simulink library "lib_all"  (Simulink 7.6)
settings: Solver=ode45  SolverMode=SingleTasking  StartTime=0.0  StopTime=10.0  RelTol=1e-3
values from neighbouring files (symbols this model uses):
  map_lib_03 = [6.8679 7.0159 7.4571 8.1849 9.1892 10.456 11.97 13.71 15.655 17.781 20.063 22.474 ... (803 elements, 73x11)]    [scr_simdata.mat]    (also in scr_simdata_all.mat)
  map_lib_06 = [2.5784 2.6727 2.9588 3.4453 4.1444 5.068 6.226 7.6242 9.2634 11.139 13.24 15.552 ... (803 elements, 73x11)]    [scr_simdata.mat]    (also in scr_simdata_all.mat)
  map_lib_09 = [0.0025784 0.0026727 0.0029588 0.0034453 0.0041444 0.005068 0.006226 0.0076242 0.0092634 0.011139 0.01324 0.015552 ... (803 elements, 73x11)]    [scr_simdata.mat]    (also in scr_simdata_all.mat)
  map_lib_10 = [2.74 2.75 2.76 2.77 2.78 2.79 2.8 2.81 2.82 2.83 2.84 2.85 ... (81 elements, 1x81)]    [scr_simdata.mat]    (also in scr_simdata_all.mat)
  map_lib_11 = [1.1983 1.2027 1.207 1.2113 1.2156 1.2198 1.2241 1.2283 1.2326 1.2368 1.241 1.2451 ... (81 elements, 1x81)]    [scr_simdata.mat]    (also in scr_simdata_all.mat)
  map_lib_12 = [2.74 2.75 2.76 2.77 2.78 2.79 2.8 2.81 2.82 2.83 2.84 2.85 ... (81 elements, 1x81)]    [scr_simdata.mat]    (also in scr_simdata_all.mat)
  map_lib_13 = [1.74 1.75 1.76 1.77 1.78 1.79 1.8 1.81 1.82 1.83 1.84 1.85 ... (81 elements, 1x81)]    [scr_simdata.mat]    (also in scr_simdata_all.mat)
  map_lib_16 = [0.00048828125 1.6159 1.8962 2.0821 2.2251 2.3426 2.4433 2.5318 2.611 2.683 2.749 2.8101 ... (1419 elements, 129x11)]    [scr_simdata.mat]    (also in scr_simdata_all.mat)
  map_mr2_01 = [0 800 1600 2400 3200 4000]    [scr_simdata.mat]    (also in scr_simdata_all.mat)
  map_mr2_02 = [1.32 1.32 1.33 1.34 1.36 1.38]    [scr_simdata.mat]    (also in scr_simdata_all.mat)
  map_mr2_03 = [0 800 1600 2400 3200 4000]    [scr_simdata.mat]    (also in scr_simdata_all.mat)
  map_mr2_04 = [1.32 1.32 1.33 1.34 1.36 1.38]    [scr_simdata.mat]    (also in scr_simdata_all.mat)
  map_mr2_05 = [0 800 1600 2400 3200 4000]    [scr_simdata.mat]    (also in scr_simdata_all.mat)
  map_mr2_06 = [1.32 1.32 1.33 1.34 1.36 1.38]    [scr_simdata.mat]    (also in scr_simdata_all.mat)
  Parameter1 = 6    [scr_simdata.mat]    (also in scr_simdata_all.mat)
  Parameter101 = 30    [scr_simdata.mat]    (also in scr_simdata_all.mat)
  Parameter102 = 12    [scr_simdata.mat]    (also in scr_simdata_all.mat)
  Parameter103 = -240    [scr_simdata.mat]    (also in scr_simdata_all.mat)
  Parameter108 = 48    [scr_simdata.mat]    (also in scr_simdata_all.mat)
  Parameter120 = 16    [scr_simdata.mat]    (also in scr_simdata_all.mat)
  Parameter121 = 87    [scr_simdata.mat]    (also in scr_simdata_all.mat)
  Parameter123 = 78    [scr_simdata.mat]    (also in scr_simdata_all.mat)
  Parameter124 = 60    [scr_simdata.mat]    (also in scr_simdata_all.mat)
  Parameter125 = -480    [scr_simdata.mat]    (also in scr_simdata_all.mat)
  Parameter130 = 96    [scr_simdata.mat]    (also in scr_simdata_all.mat)
  Parameter131 = 3    [scr_simdata.mat]    (also in scr_simdata_all.mat)
  Parameter144 = 3    [scr_simdata.mat]    (also in scr_simdata_all.mat)
  Parameter18 = 128    [scr_simdata.mat]    (also in scr_simdata_all.mat)
  Parameter19 = 16    [scr_simdata.mat]    (also in scr_simdata_all.mat)
  Parameter2 = 12    [scr_simdata.mat]    (also in scr_simdata_all.mat)
  Parameter20 = 7    [scr_simdata.mat]    (also in scr_simdata_all.mat)
  Parameter21 = 4    [scr_simdata.mat]    (also in scr_simdata_all.mat)
  Parameter3 = 18    [scr_simdata.mat]    (also in scr_simdata_all.mat)
  Parameter32 = 1    [scr_simdata.mat]    (also in scr_simdata_all.mat)
  Parameter34 = 24    [scr_simdata.mat]    (also in scr_simdata_all.mat)
  Parameter4 = 138    [scr_simdata.mat]    (also in scr_simdata_all.mat)
  Parameter42 = 48    [scr_simdata.mat]    (also in scr_simdata_all.mat)
  Parameter5 = 135    [scr_simdata.mat]    (also in scr_simdata_all.mat)
  Parameter55 = 0    [scr_simdata.mat]    (also in scr_simdata_all.mat)
  Parameter56 = 0    [scr_simdata.mat]    (also in scr_simdata_all.mat)
  Parameter57 = 1    [scr_simdata.mat]    (also in scr_simdata_all.mat)
  Parameter58 = 1    [scr_simdata.mat]    (also in scr_simdata_all.mat)
  Parameter59 = 2    [scr_simdata.mat]    (also in scr_simdata_all.mat)
  Parameter6 = 132    [scr_simdata.mat]    (also in scr_simdata_all.mat)
  Parameter60 = 2    [scr_simdata.mat]    (also in scr_simdata_all.mat)
  Parameter63 = 144    [scr_simdata.mat]    (also in scr_simdata_all.mat)
  Parameter7 = 126    [scr_simdata.mat]    (also in scr_simdata_all.mat)
  Parameter76 = 64    [scr_simdata.mat]    (also in scr_simdata_all.mat)
  Parameter77 = 135    [scr_simdata.mat]    (also in scr_simdata_all.mat)
  Parameter79 = 126    [scr_simdata.mat]    (also in scr_simdata_all.mat)
  Parameter80 = 108    [scr_simdata.mat]    (also in scr_simdata_all.mat)
  Parameter81 = 0    [scr_simdata.mat]    (also in scr_simdata_all.mat)
  Parameter86 = 0    [scr_simdata.mat]    (also in scr_simdata_all.mat)
  Parameter98 = 112    [scr_simdata.mat]    (also in scr_simdata_all.mat)
  Parameter99 = 39    [scr_simdata.mat]    (also in scr_simdata_all.mat)
undefined symbols (not defined in the file or in its folder): cylvolkp_hih_map2h, cylvolkp_map2h, pk1k_map2h
libraries: simulink x2
(part 3 of 36)

== system lib_all/SubSystem1487/SubSystem1490 ==
  note: Text291
  note: Text290
  Block4033 : Inport
  Block4034 : Inport  Port=2
  Block4035 : Inport  Port=3
  Block4036 : Constant  Value=720  OutDataTypeStr=double  SampleTime=-1
  Block4037 : Constant  Value=0  OutDataTypeStr=double  SampleTime=-1
  Block4038 : Constant  Value=0  OutDataTypeStr=double  SampleTime=-1
  Block4039 : Constant  Value=5  OutDataTypeStr=double  SampleTime=-1
  Block4040 : DataStoreRead  DataStoreName=DSM50
  Block4041 : DataStoreRead  DataStoreName=DSM35
  Block4042 : Gain  Gain=5  SaturateOnIntegerOverflow=on  OutDataTypeStr=Inherit: Same as input  ParamDataTypeStr=Inherit: Same as input
      settings: AttributesFormatString=Attribute802
  Block4043 : Gain  Gain=5  SaturateOnIntegerOverflow=on  OutDataTypeStr=Inherit: Same as input  ParamDataTypeStr=Inherit: Same as input
      settings: AttributesFormatString=Attribute803
  Block4044 : Gain  Gain=1/5  SaturateOnIntegerOverflow=on  OutDataTypeStr=Inherit: Same as input  ParamDataTypeStr=Inherit: Same as input
      settings: AttributesFormatString=Attribute804
  Block4045 : Product  Inputs=*/  OutDataTypeStr=double  SaturateOnIntegerOverflow=on  RndMeth=Zero  InputSameDT=on
  Block4046 : Rounding
  Block4047 : Rounding
  Block4048 : Sum  Inputs=+-+-  OutDataTypeStr=double  RndMeth=Zero  SaturateOnIntegerOverflow=on  InputSameDT=on
  Block4049 : Sum  Inputs=-+  OutDataTypeStr=double  RndMeth=Zero  SaturateOnIntegerOverflow=on  InputSameDT=on
  SubSystem1491 : Subsystem  TreatAsAtomicUnit=on  mask(***ATC.DIVISION***){bd_version=1.01.A, r0=チェック有り, r1=, r2=, r3=, r4=, r5=, r6=, r7=, r8=, r9=, c0=, c1=, c2=, c3=, c4=, c5=, c6=, c7=, c8=, c9=}
      mask prompts: bd_version "Version"
      mask help: これはAutoCからのブロックです．編集禁止．
      settings: OpenFcn=warndlg('変更禁止!');
      mask Help: web(ui_blockhelpmng(gcb));
  SubSystem1492 : Subsystem  TreatAsAtomicUnit=on  mask(***ATC.DSRVEC***){bd_version=1.01.A, r0=DSM95, r1=-1, r2=, r3=, r4=, r5=, r6=, r7=, r8=, r9=, c0=, c1=, c2=, c3=, c4=, c5=, c6=, c7=, c8=, c9=}
      mask prompts: bd_version "Version"
      mask help: これはAutoCからのブロックです．編集禁止．
      settings: OpenFcn=warndlg('変更禁止!');
      mask Help: web(ui_blockhelpmng(gcb));
      mask Display: disp('ATC.DSRVEC')
  SubSystem1493 : Subsystem
      settings: RTWSystemCode=Inline
  Block4058 : Outport
  Block4059 : Outport  Port=2
  wires:
  Block4047/1 -> Block4058/1
  Block4044/1 -> Block4047/1
  Block4043/1 -> Block4048/1
  Block4040/1 -> Block4048/2
  SubSystem1492/1(Output) -> SubSystem1491/1(mult)
  Block4037/1 -> SubSystem1491/3(init)
  SubSystem1491/1(Out) -> Block4048/3  "SigLabel141"
  Block4033/1 -> Block4043/1
  SubSystem1493/1(Block4057) -> Block4044/1, Block4049/2, Block4045/1
  Block4035/1 -> Block4048/4
  Block4036/1 -> SubSystem1493/1(Block4050)
  Block4048/1 -> SubSystem1493/2(Block4051)  "SigLabel140"
  Block4038/1 -> SubSystem1493/3(Block4052)
  Block4034/1 -> SubSystem1492/1(element)
  Block4039/1 -> Block4045/2
  Block4045/1 -> Block4046/1
  Block4046/1 -> Block4042/1
  Block4042/1 -> Block4049/1
  Block4049/1 -> Block4059/1
  Block4041/1 -> SubSystem1491/2(div)

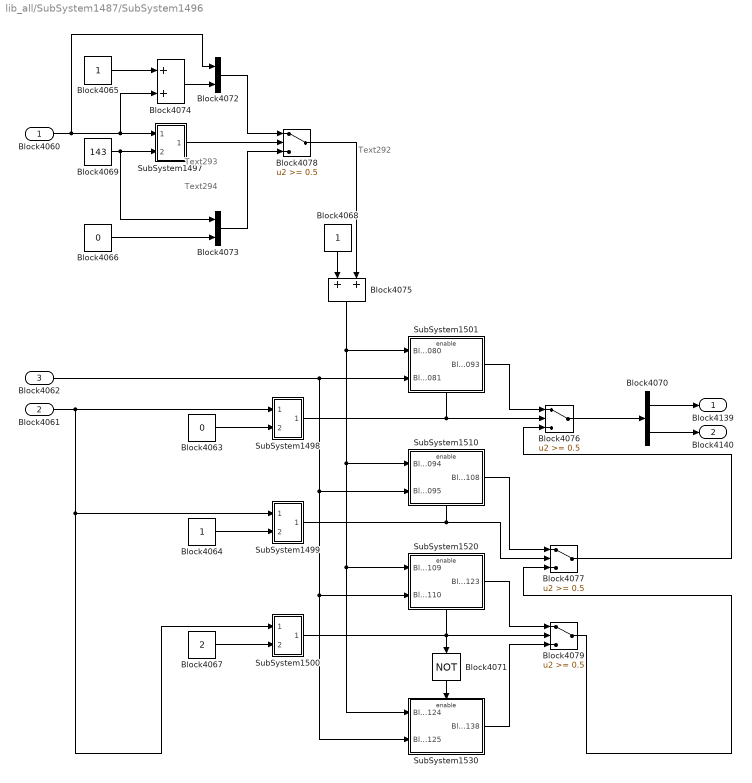
== system lib_all/SubSystem1487/SubSystem1496 ==
  note: Text294
  note: Text293
  note: Text292
  Block4060 : Inport
  Block4061 : Inport  Port=2
  Block4062 : Inport  Port=3
  Block4063 : Constant  Value=0  OutDataTypeStr=double  SampleTime=-1
  Block4064 : Constant  OutDataTypeStr=double  SampleTime=-1
  Block4065 : Constant  OutDataTypeStr=double  SampleTime=-1
  Block4066 : Constant  Value=0  OutDataTypeStr=double  SampleTime=-1
  Block4067 : Constant  Value=2  OutDataTypeStr=double  SampleTime=-1
  Block4068 : Constant  OutDataTypeStr=double  SampleTime=-1
  Block4069 : Constant  Value=143  OutDataTypeStr=double  SampleTime=-1
  Block4070 : Demux  Outputs=2
  Block4071 : Logic  Operator=NOT
  Block4072 : Mux  Inputs=2
  Block4073 : Mux  Inputs=2
  Block4074 : Sum  OutDataTypeStr=double  RndMeth=Zero  SaturateOnIntegerOverflow=on  InputSameDT=on
  Block4075 : Sum  OutDataTypeStr=double  RndMeth=Zero  SaturateOnIntegerOverflow=on  InputSameDT=on
  Block{4076..4079} (4 alike) : Switch  Threshold=0.5  RndMeth=Zero  SaturateOnIntegerOverflow=on  InputSameDT=on
  SubSystem1497 : Subsystem
  SubSystem1498 : Subsystem
  SubSystem1499 : Subsystem
  SubSystem1500 : Subsystem
  SubSystem1501 : Subsystem  TreatAsAtomicUnit=on
  SubSystem1510 : Subsystem  TreatAsAtomicUnit=on
  SubSystem1520 : Subsystem  TreatAsAtomicUnit=on
  SubSystem1530 : Subsystem  TreatAsAtomicUnit=on
  Block4139 : Outport
  Block4140 : Outport  Port=2
  wires:
  Block4078/1 -> Block4075/2
  Block4068/1 -> Block4075/1
  Block4060/1 -> SubSystem1497/1(In1), Block4074/2, Block4072/1
  Block4069/1 -> SubSystem1497/2(In2), Block4073/1
  Block4061/1 -> SubSystem1498/1(In1), SubSystem1499/1(In1), SubSystem1500/1(In1)
  Block4063/1 -> SubSystem1498/2(In2)
  SubSystem1498/1(Out1) -> Block4076/2, SubSystem1501/Enable
  SubSystem1501/1(Block4093) -> Block4076/1
  Block4064/1 -> SubSystem1499/2(In2)
  SubSystem1499/1(Out1) -> Block4077/2, SubSystem1510/Enable
  SubSystem1510/1(Block4108) -> Block4077/1
  Block4077/1 -> Block4076/3
  Block4075/1 -> SubSystem1501/1(Block4080), SubSystem1510/1(Block4094), SubSystem1520/1(Block4109), SubSystem1530/1(Block4124)
  Block4070/1 -> Block4139/1
  Block4070/2 -> Block4140/1
  Block4076/1 -> Block4070/1
  SubSystem1497/1(Out1) -> Block4078/2
  Block4065/1 -> Block4074/1
  Block4066/1 -> Block4073/2
  Block4073/1 -> Block4078/3
  Block4074/1 -> Block4072/2
  Block4072/1 -> Block4078/1
  Block4067/1 -> SubSystem1500/2(In2)
  SubSystem1500/1(Out1) -> Block4071/1, SubSystem1520/Enable, Block4079/2
  Block4071/1 -> SubSystem1530/Enable
  SubSystem1520/1(Block4123) -> Block4079/1
  SubSystem1530/1(Block4138) -> Block4079/3
  Block4079/1 -> Block4077/3
  Block4062/1 -> SubSystem1501/2(Block4081), SubSystem1510/2(Block4095), SubSystem1520/2(Block4110), SubSystem1530/2(Block4125)

== system lib_all/SubSystem1540/SubSystem1542 ==
  note: ***編集禁止***
  In1 : Inport
  In2 : Inport  Port=2
  LO1 : Logic
  RO1 : RelationalOperator  Operator=<  InputSameDT=on
  RO2 : RelationalOperator  Operator=~=  InputSameDT=on
  single1 : DataTypeConversion  OutDataTypeStr=single  SaturateOnIntegerOverflow=on  RndMeth=Zero
  single2 : DataTypeConversion  OutDataTypeStr=single  SaturateOnIntegerOverflow=on  RndMeth=Zero
  Out1 : Outport
  wires:
  LO1/1 -> Out1/1
  RO2/1 -> LO1/2
  RO1/1 -> LO1/1
  single2/1 -> RO2/2, RO1/2
  single1/1 -> RO2/1, RO1/1
  In1/1 -> single1/1
  In2/1 -> single2/1

== system lib_all/SubSystem1540/SubSystem1543 ==
  note: Text297
  note: Text296
  Block4155 : Inport
  Block4156 : Inport  Port=2
  Block4157 : Inport  Port=3
  Block4158 : Constant  Value=720  OutDataTypeStr=double  SampleTime=-1
  Block4159 : Constant  Value=0  OutDataTypeStr=double  SampleTime=-1
  Block4160 : Constant  Value=0  OutDataTypeStr=double  SampleTime=-1
  Block4161 : Constant  Value=5  OutDataTypeStr=double  SampleTime=-1
  Block4162 : DataStoreRead  DataStoreName=DSM50
  Block4163 : DataStoreRead  DataStoreName=DSM35
  Block4164 : Gain  Gain=5  SaturateOnIntegerOverflow=on  OutDataTypeStr=Inherit: Same as input  ParamDataTypeStr=Inherit: Same as input
      settings: AttributesFormatString=Attribute821
  Block4165 : Gain  Gain=5  SaturateOnIntegerOverflow=on  OutDataTypeStr=Inherit: Same as input  ParamDataTypeStr=Inherit: Same as input
      settings: AttributesFormatString=Attribute822
  Block4166 : Gain  Gain=1/5  SaturateOnIntegerOverflow=on  OutDataTypeStr=Inherit: Same as input  ParamDataTypeStr=Inherit: Same as input
      settings: AttributesFormatString=Attribute823
  Block4167 : Product  Inputs=*/  OutDataTypeStr=double  SaturateOnIntegerOverflow=on  RndMeth=Zero  InputSameDT=on
  Block4168 : Rounding
  Block4169 : Rounding
  Block4170 : Sum  Inputs=+-+-  OutDataTypeStr=double  RndMeth=Zero  SaturateOnIntegerOverflow=on  InputSameDT=on
  Block4171 : Sum  Inputs=-+  OutDataTypeStr=double  RndMeth=Zero  SaturateOnIntegerOverflow=on  InputSameDT=on
  SubSystem1544 : Subsystem  TreatAsAtomicUnit=on  mask(***ATC.DIVISION***){bd_version=1.01.A, r0=チェック有り, r1=, r2=, r3=, r4=, r5=, r6=, r7=, r8=, r9=, c0=, c1=, c2=, c3=, c4=, c5=, c6=, c7=, c8=, c9=}
      mask prompts: bd_version "Version"
      mask help: これはAutoCからのブロックです．編集禁止．
      settings: OpenFcn=warndlg('変更禁止!');
      mask Help: web(ui_blockhelpmng(gcb));
  SubSystem1545 : Subsystem  TreatAsAtomicUnit=on  mask(***ATC.DSRVEC***){bd_version=1.01.A, r0=DSM95, r1=-1, r2=, r3=, r4=, r5=, r6=, r7=, r8=, r9=, c0=, c1=, c2=, c3=, c4=, c5=, c6=, c7=, c8=, c9=}
      mask prompts: bd_version "Version"
      mask help: これはAutoCからのブロックです．編集禁止．
      settings: OpenFcn=warndlg('変更禁止!');
      mask Help: web(ui_blockhelpmng(gcb));
      mask Display: disp('ATC.DSRVEC')
  SubSystem1546 : Subsystem
      settings: RTWSystemCode=Inline
  Block4180 : Outport
  Block4181 : Outport  Port=2
  wires:
  Block4163/1 -> SubSystem1544/2(div)
  Block4171/1 -> Block4181/1
  Block4164/1 -> Block4171/1
  Block4168/1 -> Block4164/1
  Block4167/1 -> Block4168/1
  Block4161/1 -> Block4167/2
  Block4156/1 -> SubSystem1545/1(element)
  Block4160/1 -> SubSystem1546/3(Block4174)
  Block4170/1 -> SubSystem1546/2(Block4173)  "SigLabel144"
  Block4158/1 -> SubSystem1546/1(Block4172)
  Block4157/1 -> Block4170/4
  SubSystem1546/1(Block4179) -> Block4167/1, Block4171/2, Block4166/1
  Block4155/1 -> Block4165/1
  SubSystem1544/1(Out) -> Block4170/3  "SigLabel143"
  Block4159/1 -> SubSystem1544/3(init)
  SubSystem1545/1(Output) -> SubSystem1544/1(mult)
  Block4162/1 -> Block4170/2
  Block4165/1 -> Block4170/1
  Block4166/1 -> Block4169/1
  Block4169/1 -> Block4180/1

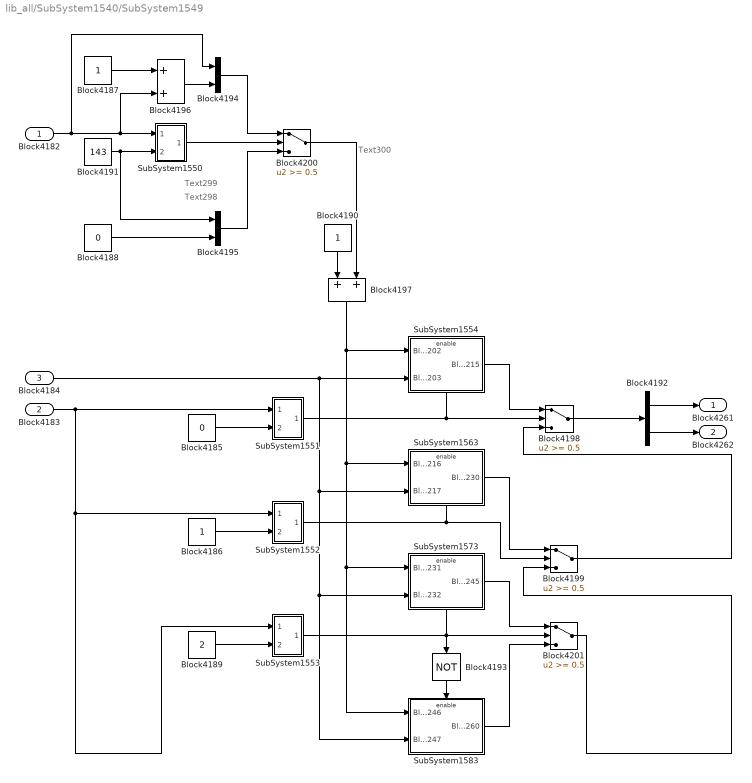
== system lib_all/SubSystem1540/SubSystem1549 ==
  note: Text300
  note: Text299
  note: Text298
  Block4182 : Inport
  Block4183 : Inport  Port=2
  Block4184 : Inport  Port=3
  Block4185 : Constant  Value=0  OutDataTypeStr=double  SampleTime=-1
  Block4186 : Constant  OutDataTypeStr=double  SampleTime=-1
  Block4187 : Constant  OutDataTypeStr=double  SampleTime=-1
  Block4188 : Constant  Value=0  OutDataTypeStr=double  SampleTime=-1
  Block4189 : Constant  Value=2  OutDataTypeStr=double  SampleTime=-1
  Block4190 : Constant  OutDataTypeStr=double  SampleTime=-1
  Block4191 : Constant  Value=143  OutDataTypeStr=double  SampleTime=-1
  Block4192 : Demux  Outputs=2
  Block4193 : Logic  Operator=NOT
  Block4194 : Mux  Inputs=2
  Block4195 : Mux  Inputs=2
  Block4196 : Sum  OutDataTypeStr=double  RndMeth=Zero  SaturateOnIntegerOverflow=on  InputSameDT=on
  Block4197 : Sum  OutDataTypeStr=double  RndMeth=Zero  SaturateOnIntegerOverflow=on  InputSameDT=on
  Block{4198..4201} (4 alike) : Switch  Threshold=0.5  RndMeth=Zero  SaturateOnIntegerOverflow=on  InputSameDT=on
  SubSystem1550 : Subsystem
  SubSystem1551 : Subsystem
  SubSystem1552 : Subsystem
  SubSystem1553 : Subsystem
  SubSystem1554 : Subsystem  TreatAsAtomicUnit=on
  SubSystem1563 : Subsystem  TreatAsAtomicUnit=on
  SubSystem1573 : Subsystem  TreatAsAtomicUnit=on
  SubSystem1583 : Subsystem  TreatAsAtomicUnit=on
  Block4261 : Outport
  Block4262 : Outport  Port=2
  wires:
  Block4184/1 -> SubSystem1583/2(Block4247), SubSystem1573/2(Block4232), SubSystem1563/2(Block4217), SubSystem1554/2(Block4203)
  Block4201/1 -> Block4199/3
  SubSystem1583/1(Block4260) -> Block4201/3
  SubSystem1573/1(Block4245) -> Block4201/1
  Block4193/1 -> SubSystem1583/Enable
  SubSystem1553/1(Out1) -> Block4201/2, SubSystem1573/Enable, Block4193/1
  Block4189/1 -> SubSystem1553/2(In2)
  Block4194/1 -> Block4200/1
  Block4196/1 -> Block4194/2
  Block4195/1 -> Block4200/3
  Block4188/1 -> Block4195/2
  Block4187/1 -> Block4196/1
  SubSystem1550/1(Out1) -> Block4200/2
  Block4198/1 -> Block4192/1
  Block4192/2 -> Block4262/1
  Block4192/1 -> Block4261/1
  Block4197/1 -> SubSystem1583/1(Block4246), SubSystem1573/1(Block4231), SubSystem1563/1(Block4216), SubSystem1554/1(Block4202)
  Block4199/1 -> Block4198/3
  SubSystem1563/1(Block4230) -> Block4199/1
  SubSystem1552/1(Out1) -> SubSystem1563/Enable, Block4199/2
  Block4186/1 -> SubSystem1552/2(In2)
  SubSystem1554/1(Block4215) -> Block4198/1
  SubSystem1551/1(Out1) -> SubSystem1554/Enable, Block4198/2
  Block4185/1 -> SubSystem1551/2(In2)
  Block4183/1 -> SubSystem1553/1(In1), SubSystem1552/1(In1), SubSystem1551/1(In1)
  Block4191/1 -> Block4195/1, SubSystem1550/2(In2)
  Block4182/1 -> Block4194/1, Block4196/2, SubSystem1550/1(In1)
  Block4190/1 -> Block4197/1
  Block4200/1 -> Block4197/2

== system lib_all/SubSystem1593/SubSystem1595 ==
  note: Text307
  Block4270 : Inport
  Block4271 : Inport  Port=2
  Block4272 : Inport  Port=3
  Block4273 : Inport  Port=4
  Block4274 : Constant  Value=0  OutDataTypeStr=double  SampleTime=-1
  Block4275 : Constant  OutDataTypeStr=double  SampleTime=-1
  Block4276 : Constant  Value=0  OutDataTypeStr=double  SampleTime=-1
  Block4277 : Gain  Gain=1/5  SaturateOnIntegerOverflow=on  OutDataTypeStr=Inherit: Same as input  ParamDataTypeStr=Inherit: Same as input
      settings: AttributesFormatString=Attribute836
  Block4278 : Product  OutDataTypeStr=double  SaturateOnIntegerOverflow=on  RndMeth=Zero  InputSameDT=on
  Block4279 : Sum  Inputs=-+  OutDataTypeStr=double  RndMeth=Zero  SaturateOnIntegerOverflow=on  InputSameDT=on
  Block4280 : Sum  OutDataTypeStr=double  RndMeth=Zero  SaturateOnIntegerOverflow=on  InputSameDT=on
  Block4281 : Sum  OutDataTypeStr=double  RndMeth=Zero  SaturateOnIntegerOverflow=on  InputSameDT=on
  Block4282 : Switch  Threshold=0.5  RndMeth=Zero  SaturateOnIntegerOverflow=on  InputSameDT=on
  SubSystem1597 : Subsystem
  SubSystem1598 : Subsystem
  SubSystem1604 : Subsystem
  Block4391 : Outport
  wires:
  Block4281/1 -> SubSystem1604/3(Block4312), SubSystem1598/2(Block4284)
  Block4270/1 -> Block4281/1
  Block4277/1 -> Block4280/2
  Block4278/1 -> Block4277/1
  Block4275/1 -> Block4281/2
  Block4274/1 -> Block4282/1
  Block4282/1 -> Block4391/1
  SubSystem1597/1(Out1) -> Block4282/2
  Block4276/1 -> SubSystem1597/2(In2)
  Block4272/1 -> SubSystem1598/3(Block4285)
  Block4280/1 -> SubSystem1597/1(In1), Block4282/3
  SubSystem1598/2(Block4309) -> Block4278/2
  Block4279/1 -> Block4278/1  "SigLabel148"
  SubSystem1604/2(Block4390) -> Block4279/2
  SubSystem1604/1(Block4389) -> Block4279/1, Block4280/1
  Block4273/1 -> SubSystem1604/2(Block4311)
  SubSystem1598/1(Block4308) -> SubSystem1604/1(Block4310)
  Block4271/1 -> SubSystem1598/1(Block4283)

== system lib_all/SubSystem1593/SubSystem1648 ==
  note: Text310
  Block4392 : Inport
  Block4393 : Inport  Port=2
  Block4394 : Inport  Port=3
  Block4395 : Inport  Port=4
  SubSystem1650 : Subsystem
  SubSystem1658 : Subsystem
  Block4437 : Outport
  wires:
  Block4394/1 -> SubSystem1650/3(Block4398)
  SubSystem1658/1(Block4436) -> Block4437/1
  Block4395/1 -> SubSystem1658/1(Block4429)
  SubSystem1650/1(Block4428) -> SubSystem1658/2(Block4430)
  Block4392/1 -> SubSystem1650/2(Block4397)
  Block4393/1 -> SubSystem1650/1(Block4396)

== system lib_all/SubSystem1662/SubSystem1664 ==
  note: Text317
  Block4458 : Inport
  Block4459 : Inport  Port=2
  Block4460 : Inport  Port=3
  Block4461 : Constant  Value=0  OutDataTypeStr=double  SampleTime=-1
  Block4462 : Constant  Value=720  OutDataTypeStr=double  SampleTime=-1
  Block4463 : Gain  Gain=5  SaturateOnIntegerOverflow=on  OutDataTypeStr=Inherit: Same as input  ParamDataTypeStr=Inherit: Same as input
      settings: AttributesFormatString=Attribute870
  Block4464 : Sum  Inputs=+-  OutDataTypeStr=double  RndMeth=Zero  SaturateOnIntegerOverflow=on  InputSameDT=on
  SubSystem1665 : Subsystem
      settings: RTWFcnNameOpts=Use subsystem name
  SubSystem1670 : Subsystem
      settings: RTWSystemCode=Inline
  Block4490 : Outport
  wires:
  Block4463/1 -> Block4464/1
  SubSystem1665/1(Block4483) -> Block4463/1
  Block4458/1 -> SubSystem1665/1(Block4465)
  Block4459/1 -> SubSystem1665/2(Block4466)
  Block4460/1 -> Block4464/2
  Block4464/1 -> SubSystem1670/2(Block4485)
  Block4462/1 -> SubSystem1670/1(Block4484)
  Block4461/1 -> SubSystem1670/3(Block4486)
  SubSystem1670/1(Block4489) -> Block4490/1

== system lib_all/SubSystem1662/SubSystem1672 ==
  note: Text318
  Block4491 : Inport
  Block4492 : Inport  Port=2
  Block4493 : Constant  Value=360  OutDataTypeStr=double  SampleTime=-1
  Block4494 : Constant  Value=360  OutDataTypeStr=double  SampleTime=-1
  Block4495 : Sum  Inputs=+-  OutDataTypeStr=double  RndMeth=Zero  SaturateOnIntegerOverflow=on  InputSameDT=on
  Block4496 : Switch  Threshold=0.5  RndMeth=Zero  SaturateOnIntegerOverflow=on  InputSameDT=on
  Block4497 : Lookup2D  RowIndex=cylvolkp_map2h_y  ColumnIndex=cylvolkp_map2h_x  Table=cylvolkp_map2h  SaturateOnIntegerOverflow=on  InputSameDT=on
      settings: AttributesFormatString=<%<OutputValues>>\nAttribute879
  SubSystem1673 : Subsystem
  Block4498 : Outport
  wires:
  Block4492/1 -> SubSystem1673/1(In1), Block4496/3, Block4495/1
  Block4494/1 -> SubSystem1673/2(In2)
  SubSystem1673/1(Out1) -> Block4496/2
  Block4495/1 -> Block4496/1
  Block4493/1 -> Block4495/2
  Block4497/1 -> Block4498/1
  Block4491/1 -> Block4497/2
  Block4496/1 -> Block4497/1  "SigLabel152"

== system lib_all/SubSystem1674/SubSystem1676 ==
  note: ***編集禁止***
  In1 : Inport
  In2 : Inport  Port=2
  RO1 : RelationalOperator  Operator===  InputSameDT=on
  single1 : DataTypeConversion  OutDataTypeStr=single  SaturateOnIntegerOverflow=on  RndMeth=Zero
  single2 : DataTypeConversion  OutDataTypeStr=single  SaturateOnIntegerOverflow=on  RndMeth=Zero
  Out1 : Outport
  wires:
  RO1/1 -> Out1/1
  In2/1 -> single2/1
  In1/1 -> single1/1
  single1/1 -> RO1/1
  single2/1 -> RO1/2

== system lib_all/SubSystem1674/SubSystem1677 ==
  note: ***編集禁止***
  In1 : Inport
  In2 : Inport  Port=2
  RO1 : RelationalOperator  Operator===  InputSameDT=on
  single1 : DataTypeConversion  OutDataTypeStr=single  SaturateOnIntegerOverflow=on  RndMeth=Zero
  single2 : DataTypeConversion  OutDataTypeStr=single  SaturateOnIntegerOverflow=on  RndMeth=Zero
  Out1 : Outport
  wires:
  RO1/1 -> Out1/1
  In2/1 -> single2/1
  In1/1 -> single1/1
  single1/1 -> RO1/1
  single2/1 -> RO1/2

== system lib_all/SubSystem1674/SubSystem1678 ==
  note: ***編集禁止***
  In1 : Inport
  In2 : Inport  Port=2
  RO1 : RelationalOperator  Operator===  InputSameDT=on
  single1 : DataTypeConversion  OutDataTypeStr=single  SaturateOnIntegerOverflow=on  RndMeth=Zero
  single2 : DataTypeConversion  OutDataTypeStr=single  SaturateOnIntegerOverflow=on  RndMeth=Zero
  Out1 : Outport
  wires:
  RO1/1 -> Out1/1
  In2/1 -> single2/1
  In1/1 -> single1/1
  single1/1 -> RO1/1
  single2/1 -> RO1/2

== system lib_all/SubSystem1674/SubSystem1679 ==
  note: ***編集禁止***
  In1 : Inport
  In2 : Inport  Port=2
  RO1 : RelationalOperator  Operator===  InputSameDT=on
  single1 : DataTypeConversion  OutDataTypeStr=single  SaturateOnIntegerOverflow=on  RndMeth=Zero
  single2 : DataTypeConversion  OutDataTypeStr=single  SaturateOnIntegerOverflow=on  RndMeth=Zero
  Out1 : Outport
  wires:
  RO1/1 -> Out1/1
  In2/1 -> single2/1
  In1/1 -> single1/1
  single1/1 -> RO1/1
  single2/1 -> RO1/2

== system lib_all/SubSystem1674/SubSystem1680 ==
  note: ***編集禁止***
  In1 : Inport
  In2 : Inport  Port=2
  RO1 : RelationalOperator  Operator===  InputSameDT=on
  single1 : DataTypeConversion  OutDataTypeStr=single  SaturateOnIntegerOverflow=on  RndMeth=Zero
  single2 : DataTypeConversion  OutDataTypeStr=single  SaturateOnIntegerOverflow=on  RndMeth=Zero
  Out1 : Outport
  wires:
  RO1/1 -> Out1/1
  In2/1 -> single2/1
  In1/1 -> single1/1
  single1/1 -> RO1/1
  single2/1 -> RO1/2

== system lib_all/SubSystem1674/SubSystem1681 ==
  note: ***編集禁止***
  In1 : Inport
  In2 : Inport  Port=2
  RO1 : RelationalOperator  Operator===  InputSameDT=on
  single1 : DataTypeConversion  OutDataTypeStr=single  SaturateOnIntegerOverflow=on  RndMeth=Zero
  single2 : DataTypeConversion  OutDataTypeStr=single  SaturateOnIntegerOverflow=on  RndMeth=Zero
  Out1 : Outport
  wires:
  RO1/1 -> Out1/1
  In2/1 -> single2/1
  In1/1 -> single1/1
  single1/1 -> RO1/1
  single2/1 -> RO1/2

== system lib_all/SubSystem1674/SubSystem1682 ==
  Block4530 : Inport
  Block4531 : Inport  Port=2
  Block4532 : Inport  Port=3
  Block4533 : EnablePort
  Block4534 : DataStoreWrite  DataStoreName=DSM20
  Block4535 : DataStoreWrite  DataStoreName=DSM30
  Block4536 : DataStoreWrite  DataStoreName=DSM25
  wires:
  Block4532/1 -> Block4536/1
  Block4531/1 -> Block4535/1
  Block4530/1 -> Block4534/1

== system lib_all/SubSystem1674/SubSystem1683 ==
  Block4537 : Inport
  Block4538 : Inport  Port=2
  Block4539 : Inport  Port=3
  Block4540 : EnablePort
  Block4541 : DataStoreWrite  DataStoreName=DSM21
  Block4542 : DataStoreWrite  DataStoreName=DSM31
  Block4543 : DataStoreWrite  DataStoreName=DSM26
  wires:
  Block4539/1 -> Block4543/1
  Block4538/1 -> Block4542/1
  Block4537/1 -> Block4541/1

== system lib_all/SubSystem1674/SubSystem1684 ==
  Block4544 : Inport
  Block4545 : Inport  Port=2
  Block4546 : Inport  Port=3
  Block4547 : EnablePort
  Block4548 : DataStoreWrite  DataStoreName=DSM22
  Block4549 : DataStoreWrite  DataStoreName=DSM32
  Block4550 : DataStoreWrite  DataStoreName=DSM27
  wires:
  Block4546/1 -> Block4550/1
  Block4545/1 -> Block4549/1
  Block4544/1 -> Block4548/1

== system lib_all/SubSystem1674/SubSystem1685 ==
  Block4551 : Inport
  Block4552 : Inport  Port=2
  Block4553 : Inport  Port=3
  Block4554 : EnablePort
  Block4555 : DataStoreWrite  DataStoreName=DSM23
  Block4556 : DataStoreWrite  DataStoreName=DSM33
  Block4557 : DataStoreWrite  DataStoreName=DSM28
  wires:
  Block4553/1 -> Block4557/1
  Block4552/1 -> Block4556/1
  Block4551/1 -> Block4555/1

== system lib_all/SubSystem1674/SubSystem1686 ==
  Block4558 : Inport
  Block4559 : Inport  Port=2
  Block4560 : Inport  Port=3
  Block4561 : EnablePort
  Block4562 : DataStoreWrite  DataStoreName=DSM24
  Block4563 : DataStoreWrite  DataStoreName=DSM34
  Block4564 : DataStoreWrite  DataStoreName=DSM29
  wires:
  Block4558/1 -> Block4562/1
  Block4559/1 -> Block4563/1
  Block4560/1 -> Block4564/1

== system lib_all/SubSystem1674/SubSystem1687 ==
  note: Text323
  note: Text322
  Block4565 : Inport
  Block4566 : Inport  Port=2
  Block4567 : Inport  Port=3
  Block4568 : Constant  Value=0  OutDataTypeStr=double  SampleTime=-1
  SubSystem1689 : Subsystem
  SubSystem1690 : Subsystem
  SubSystem1698 : Subsystem  TreatAsAtomicUnit=on
  SubSystem1699 : Subsystem
  Block4623 : Outport
  wires:
  Block4566/1 -> [TagName1], SubSystem1690/1(Block4577)
  Block4565/1 -> [TagName4], SubSystem1690/2(Block4578)
  SubSystem1690/1(Block4607) -> [TagName11], SubSystem1699/2(Block4616)
  Block4567/1 -> SubSystem1699/1(Block4615)
  SubSystem1699/1(Block4622) -> [TagName23], Block4623/1
  [TagName4] -> SubSystem1689/1(In1)
  Block4568/1 -> SubSystem1689/2(In2)
  SubSystem1689/1(Out1) -> SubSystem1698/Enable
  [TagName1] -> SubSystem1698/1(Block4608)
  [TagName11] -> SubSystem1698/2(Block4609)
  [TagName23] -> SubSystem1698/3(Block4610)

== system lib_all/SubSystem1674/SubSystem1701 ==
  note: Text331
  note: Text330
  note: Text329
  Block4624 : Inport
  Block4625 : Inport  Port=2
  Block4626 : Inport  Port=3
  Block4627 : Constant  OutDataTypeStr=double  SampleTime=-1
  Block4628 : Gain  Gain=1/5  SaturateOnIntegerOverflow=on  OutDataTypeStr=Inherit: Same as input  ParamDataTypeStr=Inherit: Same as input
      settings: AttributesFormatString=Attribute903
  Block4629 : Product  OutDataTypeStr=double  SaturateOnIntegerOverflow=on  RndMeth=Zero  InputSameDT=on
  Block4630 : Sum  Inputs=-+  OutDataTypeStr=double  RndMeth=Zero  SaturateOnIntegerOverflow=on  InputSameDT=on
  Block4631 : Sum  OutDataTypeStr=double  RndMeth=Zero  SaturateOnIntegerOverflow=on  InputSameDT=on
  Block4632 : Sum  OutDataTypeStr=double  RndMeth=Zero  SaturateOnIntegerOverflow=on  InputSameDT=on
  SubSystem1703 : Subsystem
  SubSystem1709 : Subsystem
  Block4723 : Outport
  wires:
  Block4625/1 -> SubSystem1703/1(Block4633)
  SubSystem1703/1(Block4657) -> SubSystem1709/1(Block4659)
  Block4626/1 -> SubSystem1709/2(Block4660)
  SubSystem1709/1(Block4721) -> Block4631/1, Block4630/1
  SubSystem1709/2(Block4722) -> Block4630/2
  Block4630/1 -> Block4629/1  "SigLabel156"
  SubSystem1703/2(Block4658) -> Block4629/2
  Block4631/1 -> Block4723/1
  Block4627/1 -> Block4632/2
  Block4629/1 -> Block4628/1
  Block4628/1 -> Block4631/2
  Block4624/1 -> Block4632/1
  Block4632/1 -> SubSystem1703/2(Block4634), SubSystem1709/3(Block4661)

== system lib_all/SubSystem1742/SubSystem1744 ==
  note: ***編集禁止***
  In1 : Inport
  In2 : Inport  Port=2
  RO1 : RelationalOperator  Operator===  InputSameDT=on
  single1 : DataTypeConversion  OutDataTypeStr=single  SaturateOnIntegerOverflow=on  RndMeth=Zero
  single2 : DataTypeConversion  OutDataTypeStr=single  SaturateOnIntegerOverflow=on  RndMeth=Zero
  Out1 : Outport
  wires:
  RO1/1 -> Out1/1
  In2/1 -> single2/1
  In1/1 -> single1/1
  single1/1 -> RO1/1
  single2/1 -> RO1/2

== system lib_all/SubSystem1742/SubSystem1745 ==
  note: Text339
  Block4737 : Inport
  Block4738 : Inport  Port=2
  Block4739 : Constant  Value=0  OutDataTypeStr=double  SampleTime=-1
  Block4740 : Constant  Value=720  OutDataTypeStr=double  SampleTime=-1
  Block4741 : Gain  Gain=5  SaturateOnIntegerOverflow=on  OutDataTypeStr=Inherit: Same as input  ParamDataTypeStr=Inherit: Same as input
      settings: AttributesFormatString=Attribute921
  SubSystem1746 : Subsystem
      settings: RTWFcnNameOpts=Use subsystem name
  SubSystem1751 : Subsystem
      settings: RTWSystemCode=Inline
  Block4767 : Outport
  wires:
  Block4741/1 -> SubSystem1751/2(Block4762)
  SubSystem1746/1(Block4760) -> Block4741/1
  Block4738/1 -> SubSystem1746/2(Block4743)
  Block4737/1 -> SubSystem1746/1(Block4742)
  SubSystem1751/1(Block4766) -> Block4767/1
  Block4740/1 -> SubSystem1751/1(Block4761)
  Block4739/1 -> SubSystem1751/3(Block4763)

== system lib_all/SubSystem1742/SubSystem1753 ==
  Block4768 : Inport
  Block4769 : Inport  Port=2
  Block4770 : Inport  Port=3
  Block4771 : EnablePort
  Block4772 : DataStoreWrite  DataStoreName=DSM12
  Block4773 : DataStoreWrite  DataStoreName=DSM14
  Block4774 : DataStoreWrite  DataStoreName=DSM13
  wires:
  Block4768/1 -> Block4772/1
  Block4770/1 -> Block4774/1
  Block4769/1 -> Block4773/1

== system lib_all/SubSystem1742/SubSystem1754 ==
  note: Text340
  Block4775 : Inport
  Block4776 : Inport  Port=2
  Block4777 : Constant  Value=360  OutDataTypeStr=double  SampleTime=-1
  Block4778 : Constant  Value=360  OutDataTypeStr=double  SampleTime=-1
  Block4779 : Sum  Inputs=+-  OutDataTypeStr=double  RndMeth=Zero  SaturateOnIntegerOverflow=on  InputSameDT=on
  Block4780 : Switch  Threshold=0.5  RndMeth=Zero  SaturateOnIntegerOverflow=on  InputSameDT=on
  Block4781 : Lookup2D  RowIndex=cylvolkp_hih_map2h_y  ColumnIndex=cylvolkp_hih_map2h_x  Table=cylvolkp_hih_map2h  SaturateOnIntegerOverflow=on  InputSameDT=on
      settings: AttributesFormatString=<%<OutputValues>>\nAttribute930
  SubSystem1755 : Subsystem
  Block4782 : Outport
  wires:
  Block4776/1 -> SubSystem1755/1(In1), Block4780/3, Block4779/1
  Block4778/1 -> SubSystem1755/2(In2)
  SubSystem1755/1(Out1) -> Block4780/2
  Block4779/1 -> Block4780/1
  Block4777/1 -> Block4779/2
  Block4781/1 -> Block4782/1
  Block4775/1 -> Block4781/2
  Block4780/1 -> Block4781/1  "SigLabel163"

== system lib_all/SubSystem1756/SubSystem1758 ==
  note: ***編集禁止***
  In1 : Inport
  In2 : Inport  Port=2
  RO1 : RelationalOperator  Operator===  InputSameDT=on
  single1 : DataTypeConversion  OutDataTypeStr=single  SaturateOnIntegerOverflow=on  RndMeth=Zero
  single2 : DataTypeConversion  OutDataTypeStr=single  SaturateOnIntegerOverflow=on  RndMeth=Zero
  Out1 : Outport
  wires:
  single2/1 -> RO1/2
  single1/1 -> RO1/1
  In1/1 -> single1/1
  In2/1 -> single2/1
  RO1/1 -> Out1/1

== system lib_all/SubSystem1756/SubSystem1759 ==
  note: Text343
  Block4796 : Inport
  Block4797 : Inport  Port=2
  Block4798 : Constant  Value=0  OutDataTypeStr=double  SampleTime=-1
  Block4799 : Constant  Value=720  OutDataTypeStr=double  SampleTime=-1
  Block4800 : Gain  Gain=5  SaturateOnIntegerOverflow=on  OutDataTypeStr=Inherit: Same as input  ParamDataTypeStr=Inherit: Same as input
      settings: AttributesFormatString=Attribute935
  SubSystem1760 : Subsystem
      settings: RTWFcnNameOpts=Use subsystem name
  SubSystem1765 : Subsystem
      settings: RTWSystemCode=Inline
  Block4826 : Outport
  wires:
  Block4798/1 -> SubSystem1765/3(Block4822)
  Block4799/1 -> SubSystem1765/1(Block4820)
  SubSystem1765/1(Block4825) -> Block4826/1
  Block4796/1 -> SubSystem1760/1(Block4801)
  Block4797/1 -> SubSystem1760/2(Block4802)
  SubSystem1760/1(Block4819) -> Block4800/1
  Block4800/1 -> SubSystem1765/2(Block4821)

== system lib_all/SubSystem1756/SubSystem1767 ==
  Block4827 : Inport
  Block4828 : Inport  Port=2
  Block4829 : Inport  Port=3
  Block4830 : EnablePort
  Block4831 : DataStoreWrite  DataStoreName=DSM12
  Block4832 : DataStoreWrite  DataStoreName=DSM14
  Block4833 : DataStoreWrite  DataStoreName=DSM13
  wires:
  Block4828/1 -> Block4832/1
  Block4829/1 -> Block4833/1
  Block4827/1 -> Block4831/1

== system lib_all/SubSystem1756/SubSystem1768 ==
  note: Text344
  Block4834 : Inport
  Block4835 : Inport  Port=2
  Block4836 : Constant  Value=360  OutDataTypeStr=double  SampleTime=-1
  Block4837 : Constant  Value=360  OutDataTypeStr=double  SampleTime=-1
  Block4838 : Sum  Inputs=+-  OutDataTypeStr=double  RndMeth=Zero  SaturateOnIntegerOverflow=on  InputSameDT=on
  Block4839 : Switch  Threshold=0.5  RndMeth=Zero  SaturateOnIntegerOverflow=on  InputSameDT=on
  Block4840 : Lookup2D  RowIndex=cylvolkp_hih_map2h_y  ColumnIndex=cylvolkp_hih_map2h_x  Table=cylvolkp_hih_map2h  SaturateOnIntegerOverflow=on  InputSameDT=on
      settings: AttributesFormatString=<%<OutputValues>>\nAttribute944
  SubSystem1769 : Subsystem
  Block4841 : Outport
  wires:
  Block4840/1 -> Block4841/1
  Block4836/1 -> Block4838/2
  Block4838/1 -> Block4839/1
  SubSystem1769/1(Out1) -> Block4839/2
  Block4837/1 -> SubSystem1769/2(In2)
  Block4835/1 -> Block4838/1, Block4839/3, SubSystem1769/1(In1)
  Block4834/1 -> Block4840/2
  Block4839/1 -> Block4840/1  "SigLabel164"

== system lib_all/SubSystem176/SubSystem178 ==
  Block385 : Inport
  Block386 : Constant  OutDataTypeStr=double  SampleTime=-1
  Block387 : Constant  Value=Parameter19  OutDataTypeStr=double  SampleTime=-1
  Block388 : Demux  Outputs=2
  Block389 : Sum  OutDataTypeStr=double  RndMeth=Zero  SaturateOnIntegerOverflow=on  InputSameDT=on
  SubSystem179 : Subsystem
  SubSystem180 : Subsystem
      settings: RTWSystemCode=Function  RTWFcnNameOpts=Use subsystem name
  SubSystem181 : Subsystem  TreatAsAtomicUnit=on  mask(***ATC.DSRVEC***){bd_version=1.01.A, r0=DSM378, r1=-1, r2=, r3=, r4=, r5=, r6=, r7=, r8=, r9=, c0=, c1=, c2=, c3=, c4=, c5=, c6=, c7=, c8=, c9=}
      mask prompts: bd_version "Version"
      mask help: これはAutoCからのブロックです．編集禁止．
      settings: OpenFcn=warndlg('変更禁止!');
      mask Help: web(ui_blockhelpmng(gcb));
      mask Display: disp('ATC.DSRVEC')
  SubSystem182 : Subsystem
  wires:
  Block389/1 -> SubSystem181/1(element), SubSystem180/1(Block390)
  SubSystem180/1(Block401) -> Block388/1
  Block386/1 -> Block389/2
  Block388/1 -> SubSystem182/2(Block403)  "SigLabel16"
  Block388/2 -> SubSystem182/3(Block404)  "SigLabel15"
  Block385/1 -> Block389/1, SubSystem182/1(Block402)
  SubSystem181/1(Output) -> SubSystem179/1(In1), SubSystem182/4(Block405)
  Block387/1 -> SubSystem179/2(In2)
  SubSystem179/1(Out1) -> SubSystem182/Enable

== system lib_all/SubSystem176/SubSystem303 ==
  note: Text46
  Block685 : Inport
  Block686 : Constant  Value=Parameter19  OutDataTypeStr=double  SampleTime=-1
  Block687 : Constant  OutDataTypeStr=double  SampleTime=-1
  Block688 : Sum  Inputs=|++  OutDataTypeStr=double  RndMeth=Zero  SaturateOnIntegerOverflow=on  InputSameDT=on
  SubSystem304 : Subsystem
  SubSystem305 : Subsystem
  SubSystem328 : Subsystem  TreatAsAtomicUnit=on  mask(***ATC.DSRVEC***){bd_version=1.01.A, r0=DSM378, r1=-1, r2=, r3=, r4=, r5=, r6=, r7=, r8=, r9=, c0=, c1=, c2=, c3=, c4=, c5=, c6=, c7=, c8=, c9=}
      mask prompts: bd_version "Version"
      mask help: これはAutoCからのブロックです．編集禁止．
      settings: OpenFcn=warndlg('変更禁止!');
      mask Help: web(ui_blockhelpmng(gcb));
      mask Display: disp('ATC.DSRVEC')
  wires:
  Block687/1 -> Block688/2
  Block688/1 -> SubSystem328/1(element), SubSystem305/1(Block689)
  SubSystem328/1(Output) -> SubSystem304/1(In1)
  Block685/1 -> Block688/1
  Block686/1 -> SubSystem304/2(In2)
  SubSystem304/1(Out1) -> SubSystem305/Enable

== system lib_all/SubSystem176/SubSystem329 ==
  Block735 : Constant  Value=Parameter19  OutDataTypeStr=double  SampleTime=-1
  Block736 : Constant  Value=Parameter131  OutDataTypeStr=double  SampleTime=-1
  Block737 : DataStoreRead  DataStoreName=DSM378
  Block738 : Sum  Inputs=+  OutDataTypeStr=double  RndMeth=Zero  SaturateOnIntegerOverflow=on  InputSameDT=on
  SubSystem330 : Subsystem
  SubSystem331 : Subsystem
  SubSystem332 : Subsystem  TreatAsAtomicUnit=on
  wires:
  Block737/1 -> SubSystem330/1(In1)
  Block735/1 -> SubSystem330/2(In2)
  SubSystem330/1(Out1) -> Block738/1
  Block738/1 -> SubSystem331/1(In1)
  Block736/1 -> SubSystem331/2(In2)
  SubSystem331/1(Out1) -> SubSystem332/Enable

== system lib_all/SubSystem176/SubSystem344 ==
  Block756 : Inport
  Block757 : Constant  OutDataTypeStr=double  SampleTime=-1
  Block758 : Constant  OutDataTypeStr=double  SampleTime=-1
  Block759 : Sum  Inputs=|++  OutDataTypeStr=double  RndMeth=Zero  SaturateOnIntegerOverflow=on  InputSameDT=on
  Block760 : Sum  Inputs=|++  OutDataTypeStr=double  RndMeth=Zero  SaturateOnIntegerOverflow=on  InputSameDT=on
  SubSystem345 : Subsystem  TreatAsAtomicUnit=on  mask(***ATC.DSRVEC***){bd_version=1.01.A, r0=DSM378, r1=-1, r2=, r3=, r4=, r5=, r6=, r7=, r8=, r9=, c0=, c1=, c2=, c3=, c4=, c5=, c6=, c7=, c8=, c9=}
      mask prompts: bd_version "Version"
      mask help: これはAutoCからのブロックです．編集禁止．
      settings: OpenFcn=warndlg('変更禁止!');
      mask Help: web(ui_blockhelpmng(gcb));
      mask Display: disp('ATC.DSRVEC')
  SubSystem346 : Subsystem  TreatAsAtomicUnit=on  mask(***ATC.DSWVEC***){bd_version=1.01.A, r0=DSM378, r1=-1, r2=, r3=, r4=, r5=, r6=, r7=, r8=, r9=, c0=, c1=, c2=, c3=, c4=, c5=, c6=, c7=, c8=, c9=}
      mask prompts: bd_version "Version"
      mask help: これはAutoCからのブロックです．編集禁止．
      settings: OpenFcn=warndlg('変更禁止!');
      mask Help: web(ui_blockhelpmng(gcb));
      mask Display: disp('ATC.DSRVEC')
  wires:
  Block758/1 -> Block760/2
  Block757/1 -> Block759/2
  Block756/1 -> Block760/1
  SubSystem345/1(Output) -> Block759/1
  Block760/1 -> SubSystem345/1(element), SubSystem346/2(element)
  Block759/1 -> SubSystem346/1(input)

== system lib_all/SubSystem1770/SubSystem1771 ==
  note: ***編集禁止***<<(u1g_GAJCC_ENGCYL == u1g_GAJCC_V6_1)>><<off>><<off>><<-1>>
  Constant1 : Constant  Value=(u1g_GAJCC_ENGCYL == u1g_GAJCC_V6_1)  SampleTime=-1
  EnabledSubsystem : Subsystem  TreatAsAtomicUnit=on
  # if A : Outport
  wires:
  Constant1/1 -> EnabledSubsystem/Enable
  EnabledSubsystem/1(Out1) -> # if A/1

== system lib_all/SubSystem1770/SubSystem1772 ==
  note: ***編集禁止***<<(u1g_GAJCC_ENGCYL == u1g_GAJCC_V6_2)>><<off>><<off>><<-1>>
  Constant1 : Constant  Value=(u1g_GAJCC_ENGCYL == u1g_GAJCC_V6_2)  SampleTime=-1
  EnabledSubsystem : Subsystem  TreatAsAtomicUnit=on
  # if A : Outport
  wires:
  Constant1/1 -> EnabledSubsystem/Enable
  EnabledSubsystem/1(Out1) -> # if A/1

== system lib_all/SubSystem1770/SubSystem1774 ==
  Block4843 : TriggerPort  TriggerType=function-call
  Block4844 : Constant  Value=Parameter144  OutDataTypeStr=double  SampleTime=-1
  Block4845 : Constant  Value=15  OutDataTypeStr=double  SampleTime=-1
  Block4846 : Constant  Value=63  OutDataTypeStr=double  SampleTime=-1
  Block4847 : Constant  Value=111  OutDataTypeStr=double  SampleTime=-1
  Block4848 : DataStoreRead  DataStoreName=DSM43
  Block4849 : Sum  OutDataTypeStr=double  RndMeth=Zero  SaturateOnIntegerOverflow=on  InputSameDT=on
  Block4850 : Sum  OutDataTypeStr=double  RndMeth=Zero  SaturateOnIntegerOverflow=on  InputSameDT=on
  Block4851 : Sum  OutDataTypeStr=double  RndMeth=Zero  SaturateOnIntegerOverflow=on  InputSameDT=on
  SubSystem1775 : Subsystem
  SubSystem1776 : Subsystem
  SubSystem1777 : Subsystem
  SubSystem1778 : Subsystem
      settings: RTWSystemCode=Inline
  SubSystem1791 : Subsystem
      settings: RTWSystemCode=Inline
  SubSystem1804 : Subsystem
      settings: RTWSystemCode=Inline
  wires:
  SubSystem1777/1(Out1) -> SubSystem1804/Enable
  SubSystem1776/1(Out1) -> SubSystem1791/Enable
  SubSystem1775/1(Out1) -> SubSystem1778/Enable
  Block4846/1 -> Block4849/2
  Block4847/1 -> Block4850/2
  Block4845/1 -> Block4851/2
  Block4848/1 -> SubSystem1775/1(In1), SubSystem1777/1(In1), SubSystem1776/1(In1)
  Block4851/1 -> SubSystem1777/2(In2)
  Block4850/1 -> SubSystem1776/2(In2)
  Block4849/1 -> SubSystem1775/2(In2)
  Block4844/1 -> Block4849/1, Block4851/1, Block4850/1

== system lib_all/SubSystem1770/SubSystem1817 ==
  Block4969 : TriggerPort  TriggerType=function-call
  Block4970 : Constant  Value=Parameter144  OutDataTypeStr=double  SampleTime=-1
  Block4971 : Constant  Value=39  OutDataTypeStr=double  SampleTime=-1
  Block4972 : Constant  Value=87  OutDataTypeStr=double  SampleTime=-1
  Block4973 : Constant  Value=135  OutDataTypeStr=double  SampleTime=-1
  Block4974 : DataStoreRead  DataStoreName=DSM43
  Block4975 : Sum  OutDataTypeStr=double  RndMeth=Zero  SaturateOnIntegerOverflow=on  InputSameDT=on
  Block4976 : Sum  OutDataTypeStr=double  RndMeth=Zero  SaturateOnIntegerOverflow=on  InputSameDT=on
  Block4977 : Sum  OutDataTypeStr=double  RndMeth=Zero  SaturateOnIntegerOverflow=on  InputSameDT=on
  SubSystem1818 : Subsystem
  SubSystem1819 : Subsystem
  SubSystem1820 : Subsystem
  SubSystem1821 : Subsystem
      settings: RTWSystemCode=Inline
  SubSystem1834 : Subsystem
      settings: RTWSystemCode=Inline
  SubSystem1847 : Subsystem
      settings: RTWSystemCode=Inline
  wires:
  Block4970/1 -> Block4976/1, Block4977/1, Block4975/1
  Block4975/1 -> SubSystem1818/2(In2)
  Block4976/1 -> SubSystem1819/2(In2)
  Block4977/1 -> SubSystem1820/2(In2)
  Block4974/1 -> SubSystem1819/1(In1), SubSystem1820/1(In1), SubSystem1818/1(In1)
  Block4971/1 -> Block4977/2
  Block4973/1 -> Block4976/2
  Block4972/1 -> Block4975/2
  SubSystem1818/1(Out1) -> SubSystem1821/Enable
  SubSystem1819/1(Out1) -> SubSystem1834/Enable
  SubSystem1820/1(Out1) -> SubSystem1847/Enable

== system lib_all/SubSystem1860/SubSystem1862 ==
  note: ***編集禁止***
  N : Inport
  For_loop : Stateflow chart  TreatAsAtomicUnit=on
  action : Outport
  i : Outport  Port=2
  wires:
  For_loop/2(function_call) -> action/1
  N/1 -> For_loop/1(N_Loop)
  For_loop/1(idx) -> i/1

== system lib_all/SubSystem1860/SubSystem1864 ==
  note: Text367
  Block5104 : Inport
  Block5105 : Inport  Port=2
  Block5106 : Inport  Port=3
  Block5107 : TriggerPort  TriggerType=function-call
  Block5108 : Constant  Value=0  OutDataTypeStr=double  SampleTime=-1
  Block5109 : Constant  Value=3  OutDataTypeStr=double  SampleTime=-1
  Block5110 : Constant  OutDataTypeStr=double  SampleTime=-1
  Block5111 : Constant  Value=Parameter63  OutDataTypeStr=double  SampleTime=-1
  Block5112 : Constant  OutDataTypeStr=double  SampleTime=-1
  Block5113 : Constant  OutDataTypeStr=double  SampleTime=-1
  Block5114 : Sum  Inputs=+-  OutDataTypeStr=double  RndMeth=Zero  SaturateOnIntegerOverflow=on  InputSameDT=on
  Block{5115..5119} (5 alike) : Sum  OutDataTypeStr=double  RndMeth=Zero  SaturateOnIntegerOverflow=on  InputSameDT=on
  Block5120 : Switch  Threshold=0.5  RndMeth=Zero  SaturateOnIntegerOverflow=on  InputSameDT=on
  Block5121 : Switch  Threshold=0.5  RndMeth=Zero  SaturateOnIntegerOverflow=on  InputSameDT=on
  Block5122 : UnitDelay
  SubSystem1865 : Subsystem
  SubSystem1866 : Subsystem
  SubSystem1867 : Subsystem
  Block5127 : Outport
  wires:
  Block5119/1 -> SubSystem1867/2(Block5124)
  Block5113/1 -> Block5119/2
  Block5121/1 -> Block5119/1
  Block5112/1 -> Block5118/2
  Block5104/1 -> Block5114/1, SubSystem1866/2(In2)
  Block5109/1 -> Block5114/2
  Block5114/1 -> Block5115/1
  Block5106/1 -> Block5115/2
  Block5105/1 -> Block5118/1
  Block5118/1 -> SubSystem1867/1(Block5123)
  Block5115/1 -> Block5121/3, Block5116/2, SubSystem1865/1(In1)
  Block5108/1 -> SubSystem1865/2(In2)
  Block5110/1 -> SubSystem1866/1(In1)
  SubSystem1866/1(Out1) -> Block5120/2
  SubSystem1865/1(Out1) -> Block5121/2
  Block5111/1 -> Block5116/1
  Block5116/1 -> Block5121/1
  Block5120/1 -> Block5127/1, Block5122/1
  SubSystem1867/1(Block5126) -> Block5120/1, Block5117/1
  Block5122/1 -> Block5117/2
  Block5117/1 -> Block5120/3

== system lib_all/SubSystem1871/SubSystem1872 ==
  note: ***編集禁止***
  element : Inport
  Inside_DSR : DataStoreRead  DataStoreName=DSM18
  Selector : Selector  IndexOptions=Index vector (port)  Indices=[1 3]  OutputSizes=1
  Output : Outport
  wires:
  Selector/1 -> Output/1
  element/1 -> Selector/2
  Inside_DSR/1 -> Selector/1

== system lib_all/SubSystem1871/SubSystem1873 ==
  note: ***編集禁止***
  element : Inport
  Inside_DSR : DataStoreRead  DataStoreName=DSM8
  Selector : Selector  IndexOptions=Index vector (port)  Indices=[1 3]  OutputSizes=1
  Output : Outport
  wires:
  Inside_DSR/1 -> Selector/1
  element/1 -> Selector/2
  Selector/1 -> Output/1

== system lib_all/SubSystem1871/SubSystem1874 ==
  note: ***編集禁止***
  element : Inport
  Inside_DSR : DataStoreRead  DataStoreName=DSM11
  Selector : Selector  IndexOptions=Index vector (port)  Indices=[1 3]  OutputSizes=1
  Output : Outport
  wires:
  Selector/1 -> Output/1
  element/1 -> Selector/2
  Inside_DSR/1 -> Selector/1

== system lib_all/SubSystem1875/SubSystem1876 ==
  note: ***編集禁止***
  element : Inport
  Inside_DSR : DataStoreRead  DataStoreName=DSM7
  Selector : Selector  IndexOptions=Index vector (port)  Indices=[1 3]  OutputSizes=1
  Output : Outport
  wires:
  Inside_DSR/1 -> Selector/1
  element/1 -> Selector/2
  Selector/1 -> Output/1

== system lib_all/SubSystem1875/SubSystem1877 ==
  note: ***編集禁止***
  element : Inport
  Inside_DSR : DataStoreRead  DataStoreName=DSM6
  Selector : Selector  IndexOptions=Index vector (port)  Indices=[1 3]  OutputSizes=1
  Output : Outport
  wires:
  Selector/1 -> Output/1
  element/1 -> Selector/2
  Inside_DSR/1 -> Selector/1

== system lib_all/SubSystem1875/SubSystem1878 ==
  note: ***編集禁止***
  element : Inport
  Inside_DSR : DataStoreRead  DataStoreName=DSM19
  Selector : Selector  IndexOptions=Index vector (port)  Indices=[1 3]  OutputSizes=1
  Output : Outport
  wires:
  Inside_DSR/1 -> Selector/1
  element/1 -> Selector/2
  Selector/1 -> Output/1
